simulink model "ClimaMgrForVentAndFlrDistbn"  (R2022b)
settings: Solver=FixedStepDiscrete  StartTime=0.0  StopTime=10.0  FixedStep=1  RelTol=1e-3
(part 1 of 2)
settings Model: Creator=blue.ge  LastModifiedBy=blue.ge
settings DataIOCC: SaveFormat=StructureWithTime
settings OptimizationCC: DefaultParameterBehavior=Inlined  EnhancedBackFolding=on  PassReuseOutputArgsAs=Individual arguments  ZeroExternalMemoryAtStartup=off  ZeroInternalMemoryAtStartup=off  OptimizeBlockOrder=speed  DifferentSizesBufferReuse=on  OptimizationCustomize=off  EfficientTunableParamExpr=on
settings DebuggingCC: ParameterTunabilityLossMsg=error
settings ModelReferenceCC: ModelReferenceNumInstancesAllowed=Single
settings SFSimCC: MATLABDynamicMemAlloc=off
settings RTWCC: Description=AUTOSAR  SystemTargetFile=autosar.tlc  GenCodeOnly=on  TemplateMakefile=ert_default_tmf
settings CodeAppCC: ForceParamTrailComments=on  IgnoreCustomStorageClasses=off  OperatorAnnotations=on  SimulinkDataObjDesc=on  SFDataObjDesc=on  InsertBlockDesc=on
settings STFCustomTargetCC: TargetLangStandard=C99 (ISO)  MultiwordLength=256  ParMdlRefBuildCompliant=on  CompOptLevelCompliant=on  IncludeMdlTerminateFcn=off  CombineOutputUpdateFcns=on  SuppressErrorStatus=on  ERTCustomFileBanners=on  SupportNonFinite=off  SupportComplex=off  SupportContinuousTime=off  SupportNonInlinedSFcns=off  RemoveResetFunc=on  MATLABClassNameForMDSCustomization=Simulink.SoftwareTarget.ERTCustomization  AutosarCompliant=on  UseToolchainInfoCompliant=on  LookupTableObjectStructAxisOrder=2,1,3,4,...  LUTObjectStructOrderEvenSpacing=Size,Table,Breakpoints  SystemTargetFile=autosar.tlc
settings ERTTargetCC: TargetLangStandard=C99 (ISO)  ConcurrentExecutionCompliant=off  IncludeMdlTerminateFcn=off  SuppressErrorStatus=on  SupportNonFinite=off  SupportComplex=off  ModelStepFunctionPrototypeControlCompliant=off  CPPClassGenCompliant=off  AutosarCompliant=on  LookupTableObjectStructAxisOrder=2,1,3,4,...  LUTObjectStructOrderEvenSpacing=Size,Table,Breakpoints  InlinedParameterPlacement=NonHierarchical  ERTCustomFileTemplate=example_file_process.tlc

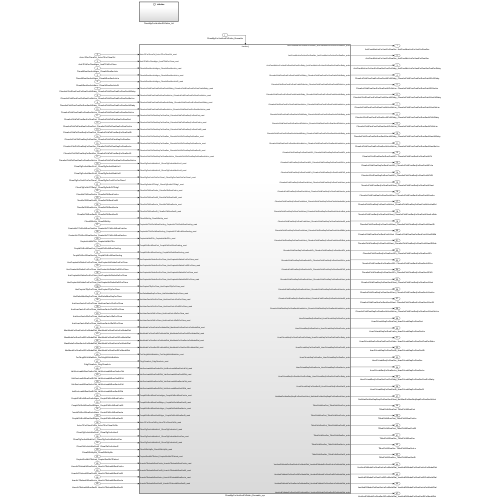
[thumbnail: Simulink's stored preview of the top-level diagram]
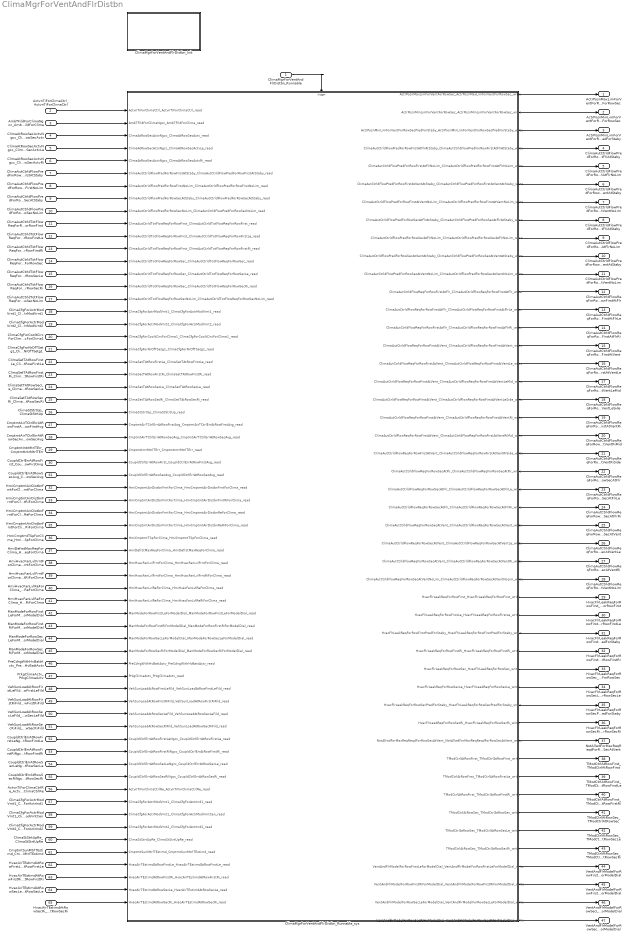
== system ClimaMgrForVentAndFlrDistbn ==
  ClimaMgrForVentAndFlrDistbn_Runnable : Inport  SampleTime=1  OutputFunctionCall=on
  ActvnTiForClimaCtrl_ActvnTiForClimaCtrl : Inport  Port=2  OutDataTypeStr=Ti33  PortDimensions=1
  AmbTFildForClimaNgcc_AmbTFildForClima : Inport  Port=3  OutDataTypeStr=T30  PortDimensions=1
  ClimaAtRowSecActvNgcc_ClimaAtRowSecActv : Inport  Port=4  OutDataTypeStr=Boolean  PortDimensions=1
  ClimaAtRowSecActvNgcc_ClimaAtRowSecActvLe : Inport  Port=5  OutDataTypeStr=Boolean  PortDimensions=1
  ClimaAtRowSecActvNgcc_ClimaAtRowSecActvRi : Inport  Port=6  OutDataTypeStr=Boolean  PortDimensions=1
  ClimaAutCtrldFlowPredForRowFirstAtStaby_ClimaAutCtrldFlowPredForRowFirstAtStaby : Inport  Port=7  OutDataTypeStr=AirFlow30  PortDimensions=1
  ClimaAutCtrldFlowPredForRowFirstNoLim_ClimaAutCtrldFlowPredForRowFirstNoLim : Inport  Port=8  OutDataTypeStr=AirFlow30  PortDimensions=1
  ClimaAutCtrldFlowPredForRowSecAtStaby_ClimaAutCtrldFlowPredForRowSecAtStaby : Inport  Port=9  OutDataTypeStr=AirFlow30  PortDimensions=1
  ClimaAutCtrldFlowPredForRowSecNoLim_ClimaAutCtrldFlowPredForRowSecNoLim : Inport  Port=10  OutDataTypeStr=AirFlow30  PortDimensions=1
  ClimaAutCtrldTotFlowReqForRowFirst_ClimaAutCtrldTotFlowReqForRowFirst : Inport  Port=11  OutDataTypeStr=AirFlow30  PortDimensions=1
  ClimaAutCtrldTotFlowReqForRowFirst_ClimaAutCtrldTotFlowReqForRowFirstLe : Inport  Port=12  OutDataTypeStr=AirFlow30  PortDimensions=1
  ClimaAutCtrldTotFlowReqForRowFirst_ClimaAutCtrldTotFlowReqForRowFirstRi : Inport  Port=13  OutDataTypeStr=AirFlow30  PortDimensions=1
  ClimaAutCtrldTotFlowReqForRowSec_ClimaAutCtrldTotFlowReqForRowSec : Inport  Port=14  OutDataTypeStr=AirFlow30  PortDimensions=1
  ClimaAutCtrldTotFlowReqForRowSec_ClimaAutCtrldTotFlowReqForRowSecLe : Inport  Port=15  OutDataTypeStr=AirFlow30  PortDimensions=1
  ClimaAutCtrldTotFlowReqForRowSec_ClimaAutCtrldTotFlowReqForRowSecRi : Inport  Port=16  OutDataTypeStr=AirFlow30  PortDimensions=1
  ClimaAutCtrldTotFlowReqForRowSecNoLim_ClimaAutCtrldTotFlowReqForRowSecNoLim : Inport  Port=17  OutDataTypeStr=AirFlow30  PortDimensions=1
  ClimaCfgForActrModVrnt1_ClimaCfgForActrModVrnt1 : Inport  Port=18  OutDataTypeStr=Enum: ClimaCfgForActrModVrnt1  PortDimensions=1
  ClimaCfgForActrModVrnt2_ClimaCfgForActrModVrnt2 : Inport  Port=19  OutDataTypeStr=Enum: ClimaCfgForActrModVrnt2  PortDimensions=1
  ClimaCfgForCooltCircForClima1_ClimaCfgForCooltCircForClima1 : Inport  Port=20  OutDataTypeStr=Boolean  PortDimensions=1
  ClimaCfgForNrOfTSetg1_ClimaCfgForNrOfTSetg1 : Inport  Port=21  OutDataTypeStr=Enum: ClimaCfgForNrOfTSetg1  PortDimensions=1
  ClimaSetTAtRowFirstLe_ClimaSetTAtRowFirstLe : Inport  Port=22  OutDataTypeStr=T30  PortDimensions=1
  ClimaSetTAtRowFirstRi_ClimaSetTAtRowFirstRi : Inport  Port=23  OutDataTypeStr=T30  PortDimensions=1
  ClimaSetTAtRowSecLe_ClimaSetTAtRowSecLe : Inport  Port=24  OutDataTypeStr=T30  PortDimensions=1
  ClimaSetTAtRowSecRi_ClimaSetTAtRowSecRi : Inport  Port=25  OutDataTypeStr=T30  PortDimensions=1
  ClimaStStrtUp_ClimaStStrtUp : Inport  Port=26  OutDataTypeStr=Enum: ClimaStStrtUp  PortDimensions=1
  CmptmtAirTCtrlErrAtRowFirstAvg_CmptmtAirTCtrlErrAtRowFirstAvg : Inport  Port=27  OutDataTypeStr=T30  PortDimensions=1
  CmptmtAirTCtrlErrAtRowSecAvg_CmptmtAirTCtrlErrAtRowSecAvg : Inport  Port=28  OutDataTypeStr=T30  PortDimensions=1
  CmptmtIntrMtrlTErr_CmptmtIntrMtrlTErr : Inport  Port=29  OutDataTypeStr=T30  PortDimensions=1
  CoupldCtrlErrAtRowFirst_CoupldCtrlErrAtRowFirstAvg : Inport  Port=30  OutDataTypeStr=T30  PortDimensions=1
  CoupldCtrlErrAtRowSecAvg_CoupldCtrlErrAtRowSecAvg : Inport  Port=31  OutDataTypeStr=T30  PortDimensions=1
  HmiCmptmtAirDistbnFrntForClima_HmiCmptmtAirDistbnFrntForClima : Inport  Port=32  OutDataTypeStr=Enum: HmiCmptmtAirDistbnFrnt  PortDimensions=1
  HmiCmptmtAirDistbnFrntForClima_HmiCmptmtAirDistbnFrntRiForClima : Inport  Port=33  OutDataTypeStr=Enum: HmiCmptmtAirDistbnFrnt  PortDimensions=1
  HmiCmptmtAirDistbnFrntForClima_HmiCmptmtAirDistbnReForClima : Inport  Port=34  OutDataTypeStr=Enum: HmiCmptmtAirDistbnFrnt  PortDimensions=1
  HmiCmptmtAirDistbnFrntForClima_HmiCmptmtAirDistbnReRiForClima : Inport  Port=35  OutDataTypeStr=Enum: HmiCmptmtAirDistbnFrnt  PortDimensions=1
  HmiCmptmtTSpForClima_HmiCmptmtTSpForClima : Inport  Port=36  OutDataTypeStr=Bus: HmiCmptmtTSp  PortDimensions=1  BusOutputAsStruct=on
  HmiDefrstMaxReqForClima_HmiDefrstMaxReqForClima : Inport  Port=37  OutDataTypeStr=Enum: ActrReq  PortDimensions=1
  HmiHvacFanLvlFrntForClima_HmiHvacFanLvlFrntForClima : Inport  Port=38  OutDataTypeStr=Enum: HmiHvacFanLvl  PortDimensions=1
  HmiHvacFanLvlFrntForClima_HmiHvacFanLvlFrntRiForClima : Inport  Port=39  OutDataTypeStr=Enum: HmiHvacFanLvl  PortDimensions=1
  HmiHvacFanLvlReForClima_HmiHvacFanLvlReForClima : Inport  Port=40  OutDataTypeStr=Enum: HmiHvacFanLvl  PortDimensions=1
  HmiHvacFanLvlReForClima_HmiHvacFanLvlReRiForClima : Inport  Port=41  OutDataTypeStr=Enum: HmiHvacFanLvl  PortDimensions=1
  ManModeForRowFirstLeForModelDial_ManModeForRowFirstLeForModelDial : Inport  Port=42  OutDataTypeStr=Enum: HmiCmptmtAirDistbnFrnt  PortDimensions=1
  ManModeForRowFirstRiForModelDial_ManModeForRowFirstRiForModelDial : Inport  Port=43  OutDataTypeStr=Enum: HmiCmptmtAirDistbnFrnt  PortDimensions=1
  ManModeForRowSecLeForModelDial_ManModeForRowSecLeForModelDial : Inport  Port=44  OutDataTypeStr=Enum: HmiCmptmtAirDistbnFrnt  PortDimensions=1
  ManModeForRowSecRiForModelDial_ManModeForRowSecRiForModelDial : Inport  Port=45  OutDataTypeStr=Enum: HmiCmptmtAirDistbnFrnt  PortDimensions=1
  PreCdngWithHvBattActv_PreCdngWithHvBattActv : Inport  Port=46  OutDataTypeStr=Boolean  PortDimensions=1
  PrkgClimaActv_PrkgClimaActv : Inport  Port=47  OutDataTypeStr=Enum: PrkgClimaActv  PortDimensions=1
  VehSunLoadAtRowFirstLeFild_VehSunLoadAtRowFirstLeFild : Inport  Port=48  OutDataTypeStr=SunLoad30  PortDimensions=1
  VehSunLoadAtRowFirstRiFild_VehSunLoadAtRowFirstRiFild : Inport  Port=49  OutDataTypeStr=SunLoad30  PortDimensions=1
  VehSunLoadAtRowSecLeFild_VehSunLoadAtRowSecLeFild : Inport  Port=50  OutDataTypeStr=SunLoad30  PortDimensions=1
  VehSunLoadAtRowSecRiFild_VehSunLoadAtRowSecRiFild : Inport  Port=51  OutDataTypeStr=SunLoad30  PortDimensions=1
  CoupldCtrlErrAtRowFirstLeNgcc_CoupldCtrlErrAtRowFirstLe : Inport  Port=52  OutDataTypeStr=T30  PortDimensions=1
  CoupldCtrlErrAtRowFirstRiNgcc_CoupldCtrlErrAtRowFirstRi : Inport  Port=53  OutDataTypeStr=T30  PortDimensions=1
  CoupldCtrlErrAtRowSecLeNgcc_CoupldCtrlErrAtRowSecLe : Inport  Port=54  OutDataTypeStr=T30  PortDimensions=1
  CoupldCtrlErrAtRowSecRiNgcc_CoupldCtrlErrAtRowSecRi : Inport  Port=55  OutDataTypeStr=T30  PortDimensions=1
  ActvnTiForClimaCtrlRe_ActvnTiForClimaCtrlRe : Inport  Port=56  OutDataTypeStr=Ti33  PortDimensions=1
  ClimaCfgForActrModVrnt1_ClimaCfgForActrInd1 : Inport  Port=57  OutDataTypeStr=Enum: ClimaCfgForActrInd1  PortDimensions=1
  ClimaCfgForActrModVrnt1_ClimaCfgForActrModVrntCen : Inport  Port=58  OutDataTypeStr=Enum: ClimaCfgForActrModVrntCen  PortDimensions=1
  ClimaCfgForActrModVrnt2_ClimaCfgForActrInd2 : Inport  Port=59  OutDataTypeStr=Enum: ClimaCfgForActrInd2  PortDimensions=1
  ClimaStStrtUpRe_ClimaStStrtUpRe : Inport  Port=60  OutDataTypeStr=Enum: ClimaStStrtUp  PortDimensions=1
  CmptmtSunMtrlTEstimd_CmptmtSunMtrlTEstimd : Inport  Port=61  OutDataTypeStr=T30  PortDimensions=1
  HvacAirTEstimdAtRowFirstLe_HvacAirTEstimdAtRowFirstLe : Inport  Port=62  OutDataTypeStr=T30  PortDimensions=1
  HvacAirTEstimdAtRowFirstRi_HvacAirTEstimdAtRowFirstRi : Inport  Port=63  OutDataTypeStr=T30  PortDimensions=1
  HvacAirTEstimdAtRowSecLe_HvacAirTEstimdAtRowSecLe : Inport  Port=64  OutDataTypeStr=T30  PortDimensions=1
  HvacAirTEstimdAtRowSecRi_HvacAirTEstimdAtRowSecRi : Inport  Port=65  OutDataTypeStr=T30  PortDimensions=1
  ClimaMgrForVentAndFlrDistbn_Init : Subsystem
  ClimaMgrForVentAndFlrDistbn_Runnable_sys : Subsystem
  ActrPosnMaxLimForVentForRowSec_ActrPosnMaxLimForVentForRowSec : Outport  OutDataTypeStr=Perc30  PortDimensions=1
  ActrPosnMinLimForVentForRowSec_ActrPosnMinLimForVentForRowSec : Outport  Port=2  OutDataTypeStr=Perc30  PortDimensions=1
  ActrPosnMinLimForVentForRowSecPredForStaby_ActrPosnMinLimForVentForRowSecPredForStaby : Outport  Port=3  OutDataTypeStr=Perc30  PortDimensions=1
  ClimaAutCtrldFlowPredForRowFirstAtFlrAtStaby_ClimaAutCtrldFlowPredForRowFirstAtFlrAtStaby : Outport  Port=4  OutDataTypeStr=AirFlow30  PortDimensions=1
  ClimaAutCtrldFlowPredForRowFirstAtFlrNoLim_ClimaAutCtrldFlowPredForRowFirstAtFlrNoLim : Outport  Port=5  OutDataTypeStr=AirFlow30  PortDimensions=1
  ClimaAutCtrldFlowPredForRowFirstAtVentAtStaby_ClimaAutCtrldFlowPredForRowFirstAtVentAtStaby : Outport  Port=6  OutDataTypeStr=AirFlow30  PortDimensions=1
  ClimaAutCtrldFlowPredForRowFirstAtVentNoLim_ClimaAutCtrldFlowPredForRowFirstAtVentNoLim : Outport  Port=7  OutDataTypeStr=AirFlow30  PortDimensions=1
  ClimaAutCtrldFlowPredForRowSecAtFlrAtStaby_ClimaAutCtrldFlowPredForRowSecAtFlrAtStaby : Outport  Port=8  OutDataTypeStr=AirFlow30  PortDimensions=1
  ClimaAutCtrldFlowPredForRowSecAtFlrNoLim_ClimaAutCtrldFlowPredForRowSecAtFlrNoLim : Outport  Port=9  OutDataTypeStr=AirFlow30  PortDimensions=1
  ClimaAutCtrldFlowPredForRowSecAtVentAtStaby_ClimaAutCtrldFlowPredForRowSecAtVentAtStaby : Outport  Port=10  OutDataTypeStr=AirFlow30  PortDimensions=1
  ClimaAutCtrldFlowPredForRowSecAtVentNoLim_ClimaAutCtrldFlowPredForRowSecAtVentNoLim : Outport  Port=11  OutDataTypeStr=AirFlow30  PortDimensions=1
  ClimaAutCtrldFlowReqForRowFirstAtFlr_ClimaAutCtrldFlowReqForRowFirstAtFlr : Outport  Port=12  OutDataTypeStr=AirFlow30  PortDimensions=1
  ClimaAutCtrldFlowReqForRowFirstAtFlr_ClimaAutCtrldFlowReqForRowFirstAtFlrLe : Outport  Port=13  OutDataTypeStr=AirFlow30  PortDimensions=1
  ClimaAutCtrldFlowReqForRowFirstAtFlr_ClimaAutCtrldFlowReqForRowFirstAtFlrRi : Outport  Port=14  OutDataTypeStr=AirFlow30  PortDimensions=1
  ClimaAutCtrldFlowReqForRowFirstAtVent_ClimaAutCtrldFlowReqForRowFirstAtVent : Outport  Port=15  OutDataTypeStr=AirFlow30  PortDimensions=1
  ClimaAutCtrldFlowReqForRowFirstAtVent_ClimaAutCtrldFlowReqForRowFirstAtVentLe : Outport  Port=16  OutDataTypeStr=AirFlow30  PortDimensions=1
  ClimaAutCtrldFlowReqForRowFirstAtVent_ClimaAutCtrldFlowReqForRowFirstAtVentLeMid : Outport  Port=17  OutDataTypeStr=AirFlow30  PortDimensions=1
  ClimaAutCtrldFlowReqForRowFirstAtVent_ClimaAutCtrldFlowReqForRowFirstAtVentLeSide : Outport  Port=18  OutDataTypeStr=AirFlow30  PortDimensions=1
  ClimaAutCtrldFlowReqForRowFirstAtVent_ClimaAutCtrldFlowReqForRowFirstAtVentRi : Outport  Port=19  OutDataTypeStr=AirFlow30  PortDimensions=1
  ClimaAutCtrldFlowReqForRowFirstAtVent_ClimaAutCtrldFlowReqForRowFirstAtVentRiMid : Outport  Port=20  OutDataTypeStr=AirFlow30  PortDimensions=1
  ClimaAutCtrldFlowReqForRowFirstAtVent_ClimaAutCtrldFlowReqForRowFirstAtVentRiSide : Outport  Port=21  OutDataTypeStr=AirFlow30  PortDimensions=1
  ClimaAutCtrldFlowReqForRowSecAtFlr_ClimaAutCtrldFlowReqForRowSecAtFlr : Outport  Port=22  OutDataTypeStr=AirFlow30  PortDimensions=1
  ClimaAutCtrldFlowReqForRowSecAtFlr_ClimaAutCtrldFlowReqForRowSecAtFlrLe : Outport  Port=23  OutDataTypeStr=AirFlow30  PortDimensions=1
  ClimaAutCtrldFlowReqForRowSecAtFlr_ClimaAutCtrldFlowReqForRowSecAtFlrRi : Outport  Port=24  OutDataTypeStr=AirFlow30  PortDimensions=1
  ClimaAutCtrldFlowReqForRowSecAtVent_ClimaAutCtrldFlowReqForRowSecAtVent : Outport  Port=25  OutDataTypeStr=AirFlow30  PortDimensions=1
  ClimaAutCtrldFlowReqForRowSecAtVent_ClimaAutCtrldFlowReqForRowSecAtVentLe : Outport  Port=26  OutDataTypeStr=AirFlow30  PortDimensions=1
  ClimaAutCtrldFlowReqForRowSecAtVent_ClimaAutCtrldFlowReqForRowSecAtVentRi : Outport  Port=27  OutDataTypeStr=AirFlow30  PortDimensions=1
  ClimaAutCtrldFlowReqForRowSecAtVentNoLim_ClimaAutCtrldFlowReqForRowSecAtVentNoLim : Outport  Port=28  OutDataTypeStr=AirFlow30  PortDimensions=1
  HvacFlrLeakReqForRowFirst_HvacFlrLeakReqForRowFirst : Outport  Port=29  OutDataTypeStr=Boolean  PortDimensions=1
  HvacFlrLeakReqForRowFirstLe_HvacFlrLeakReqForRowFirstLe : Outport  Port=30  OutDataTypeStr=Boolean  PortDimensions=1
  HvacFlrLeakReqForRowFirstPredForStaby_HvacFlrLeakReqForRowFirstPredForStaby : Outport  Port=31  OutDataTypeStr=Boolean  PortDimensions=1
  HvacFlrLeakReqForRowFirstRi_HvacFlrLeakReqForRowFirstRi : Outport  Port=32  OutDataTypeStr=Boolean  PortDimensions=1
  HvacFlrLeakReqForRowSec_HvacFlrLeakReqForRowSec : Outport  Port=33  OutDataTypeStr=Boolean  PortDimensions=1
  HvacFlrLeakReqForRowSecLe_HvacFlrLeakReqForRowSecLe : Outport  Port=34  OutDataTypeStr=Boolean  PortDimensions=1
  HvacFlrLeakReqForRowSecPredForStaby_HvacFlrLeakReqForRowSecPredForStaby : Outport  Port=35  OutDataTypeStr=Boolean  PortDimensions=1
  HvacFlrLeakReqForRowSecRi_HvacFlrLeakReqForRowSecRi : Outport  Port=36  OutDataTypeStr=Boolean  PortDimensions=1
  NotAllwdForMaxReqReqdForRowSecAtVent_NotAllwdForMaxReqReqdForRowSecAtVent : Outport  Port=37  OutDataTypeStr=Boolean  PortDimensions=1
  TModCtrlAtRowFirst_TModCtrlAtRowFirst : Outport  Port=38  OutDataTypeStr=T31  PortDimensions=1
  TModCtrlAtRowFirst_TModCtrlAtRowFirstLe : Outport  Port=39  OutDataTypeStr=T31  PortDimensions=1
  TModCtrlAtRowFirst_TModCtrlAtRowFirstRi : Outport  Port=40  OutDataTypeStr=T31  PortDimensions=1
  TModCtrlAtRowSec_TModCtrlAtRowSec : Outport  Port=41  OutDataTypeStr=T31  PortDimensions=1
  TModCtrlAtRowSec_TModCtrlAtRowSecLe : Outport  Port=42  OutDataTypeStr=T31  PortDimensions=1
  TModCtrlAtRowSec_TModCtrlAtRowSecRi : Outport  Port=43  OutDataTypeStr=T31  PortDimensions=1
  VentAndFlrModelForRowFirstLeForModelDial_VentAndFlrModelForRowFirstLeForModelDial : Outport  Port=44  OutDataTypeStr=Enum: HmiCmptmtAirDistbnFrnt  PortDimensions=1
  VentAndFlrModelForRowFirstRiForModelDial_VentAndFlrModelForRowFirstRiForModelDial : Outport  Port=45  OutDataTypeStr=Enum: HmiCmptmtAirDistbnFrnt  PortDimensions=1
  VentAndFlrModelForRowSecLeForModelDial_VentAndFlrModelForRowSecLeForModelDial : Outport  Port=46  OutDataTypeStr=Enum: HmiCmptmtAirDistbnFrnt  PortDimensions=1
  VentAndFlrModelForRowSecRiForModelDial_VentAndFlrModelForRowSecRiForModelDial : Outport  Port=47  OutDataTypeStr=Enum: HmiCmptmtAirDistbnFrnt  PortDimensions=1
  wires:
  ActvnTiForClimaCtrl_ActvnTiForClimaCtrl/1 -> ClimaMgrForVentAndFlrDistbn_Runnable_sys/1(ActvnTiForClimaCtrl_ActvnTiForClimaCtrl_read)
  AmbTFildForClimaNgcc_AmbTFildForClima/1 -> ClimaMgrForVentAndFlrDistbn_Runnable_sys/2(AmbTFildForClimaNgcc_AmbTFildForClima_read)
  ClimaAtRowSecActvNgcc_ClimaAtRowSecActv/1 -> ClimaMgrForVentAndFlrDistbn_Runnable_sys/3(ClimaAtRowSecActvNgcc_ClimaAtRowSecActv_read)
  ClimaAtRowSecActvNgcc_ClimaAtRowSecActvLe/1 -> ClimaMgrForVentAndFlrDistbn_Runnable_sys/4(ClimaAtRowSecActvNgcc_ClimaAtRowSecActvLe_read)
  ClimaAtRowSecActvNgcc_ClimaAtRowSecActvRi/1 -> ClimaMgrForVentAndFlrDistbn_Runnable_sys/5(ClimaAtRowSecActvNgcc_ClimaAtRowSecActvRi_read)
  ClimaAutCtrldFlowPredForRowFirstAtStaby_ClimaAutCtrldFlowPredForRowFirstAtStaby/1 -> ClimaMgrForVentAndFlrDistbn_Runnable_sys/6(ClimaAutCtrldFlowPredForRowFirstAtStaby_ClimaAutCtrldFlowPredForRowFirstAtStaby_read)
  ClimaAutCtrldFlowPredForRowFirstNoLim_ClimaAutCtrldFlowPredForRowFirstNoLim/1 -> ClimaMgrForVentAndFlrDistbn_Runnable_sys/7(ClimaAutCtrldFlowPredForRowFirstNoLim_ClimaAutCtrldFlowPredForRowFirstNoLim_read)
  ClimaAutCtrldFlowPredForRowSecAtStaby_ClimaAutCtrldFlowPredForRowSecAtStaby/1 -> ClimaMgrForVentAndFlrDistbn_Runnable_sys/8(ClimaAutCtrldFlowPredForRowSecAtStaby_ClimaAutCtrldFlowPredForRowSecAtStaby_read)
  ClimaAutCtrldFlowPredForRowSecNoLim_ClimaAutCtrldFlowPredForRowSecNoLim/1 -> ClimaMgrForVentAndFlrDistbn_Runnable_sys/9(ClimaAutCtrldFlowPredForRowSecNoLim_ClimaAutCtrldFlowPredForRowSecNoLim_read)
  ClimaAutCtrldTotFlowReqForRowFirst_ClimaAutCtrldTotFlowReqForRowFirst/1 -> ClimaMgrForVentAndFlrDistbn_Runnable_sys/10(ClimaAutCtrldTotFlowReqForRowFirst_ClimaAutCtrldTotFlowReqForRowFirst_read)
  ClimaAutCtrldTotFlowReqForRowFirst_ClimaAutCtrldTotFlowReqForRowFirstLe/1 -> ClimaMgrForVentAndFlrDistbn_Runnable_sys/11(ClimaAutCtrldTotFlowReqForRowFirst_ClimaAutCtrldTotFlowReqForRowFirstLe_read)
  ClimaAutCtrldTotFlowReqForRowFirst_ClimaAutCtrldTotFlowReqForRowFirstRi/1 -> ClimaMgrForVentAndFlrDistbn_Runnable_sys/12(ClimaAutCtrldTotFlowReqForRowFirst_ClimaAutCtrldTotFlowReqForRowFirstRi_read)
  ClimaAutCtrldTotFlowReqForRowSec_ClimaAutCtrldTotFlowReqForRowSec/1 -> ClimaMgrForVentAndFlrDistbn_Runnable_sys/13(ClimaAutCtrldTotFlowReqForRowSec_ClimaAutCtrldTotFlowReqForRowSec_read)
  ClimaAutCtrldTotFlowReqForRowSec_ClimaAutCtrldTotFlowReqForRowSecLe/1 -> ClimaMgrForVentAndFlrDistbn_Runnable_sys/14(ClimaAutCtrldTotFlowReqForRowSec_ClimaAutCtrldTotFlowReqForRowSecLe_read)
  ClimaAutCtrldTotFlowReqForRowSec_ClimaAutCtrldTotFlowReqForRowSecRi/1 -> ClimaMgrForVentAndFlrDistbn_Runnable_sys/15(ClimaAutCtrldTotFlowReqForRowSec_ClimaAutCtrldTotFlowReqForRowSecRi_read)
  ClimaAutCtrldTotFlowReqForRowSecNoLim_ClimaAutCtrldTotFlowReqForRowSecNoLim/1 -> ClimaMgrForVentAndFlrDistbn_Runnable_sys/16(ClimaAutCtrldTotFlowReqForRowSecNoLim_ClimaAutCtrldTotFlowReqForRowSecNoLim_read)
  ClimaCfgForActrModVrnt1_ClimaCfgForActrModVrnt1/1 -> ClimaMgrForVentAndFlrDistbn_Runnable_sys/17(ClimaCfgForActrModVrnt1_ClimaCfgForActrModVrnt1_read)
  ClimaCfgForActrModVrnt2_ClimaCfgForActrModVrnt2/1 -> ClimaMgrForVentAndFlrDistbn_Runnable_sys/18(ClimaCfgForActrModVrnt2_ClimaCfgForActrModVrnt2_read)
  ClimaCfgForCooltCircForClima1_ClimaCfgForCooltCircForClima1/1 -> ClimaMgrForVentAndFlrDistbn_Runnable_sys/19(ClimaCfgForCooltCircForClima1_ClimaCfgForCooltCircForClima1_read)
  ClimaCfgForNrOfTSetg1_ClimaCfgForNrOfTSetg1/1 -> ClimaMgrForVentAndFlrDistbn_Runnable_sys/20(ClimaCfgForNrOfTSetg1_ClimaCfgForNrOfTSetg1_read)
  ClimaSetTAtRowFirstLe_ClimaSetTAtRowFirstLe/1 -> ClimaMgrForVentAndFlrDistbn_Runnable_sys/21(ClimaSetTAtRowFirstLe_ClimaSetTAtRowFirstLe_read)
  ClimaSetTAtRowFirstRi_ClimaSetTAtRowFirstRi/1 -> ClimaMgrForVentAndFlrDistbn_Runnable_sys/22(ClimaSetTAtRowFirstRi_ClimaSetTAtRowFirstRi_read)
  ClimaSetTAtRowSecLe_ClimaSetTAtRowSecLe/1 -> ClimaMgrForVentAndFlrDistbn_Runnable_sys/23(ClimaSetTAtRowSecLe_ClimaSetTAtRowSecLe_read)
  ClimaSetTAtRowSecRi_ClimaSetTAtRowSecRi/1 -> ClimaMgrForVentAndFlrDistbn_Runnable_sys/24(ClimaSetTAtRowSecRi_ClimaSetTAtRowSecRi_read)
  ClimaStStrtUp_ClimaStStrtUp/1 -> ClimaMgrForVentAndFlrDistbn_Runnable_sys/25(ClimaStStrtUp_ClimaStStrtUp_read)
  CmptmtAirTCtrlErrAtRowFirstAvg_CmptmtAirTCtrlErrAtRowFirstAvg/1 -> ClimaMgrForVentAndFlrDistbn_Runnable_sys/26(CmptmtAirTCtrlErrAtRowFirstAvg_CmptmtAirTCtrlErrAtRowFirstAvg_read)
  CmptmtAirTCtrlErrAtRowSecAvg_CmptmtAirTCtrlErrAtRowSecAvg/1 -> ClimaMgrForVentAndFlrDistbn_Runnable_sys/27(CmptmtAirTCtrlErrAtRowSecAvg_CmptmtAirTCtrlErrAtRowSecAvg_read)
  CmptmtIntrMtrlTErr_CmptmtIntrMtrlTErr/1 -> ClimaMgrForVentAndFlrDistbn_Runnable_sys/28(CmptmtIntrMtrlTErr_CmptmtIntrMtrlTErr_read)
  CoupldCtrlErrAtRowFirst_CoupldCtrlErrAtRowFirstAvg/1 -> ClimaMgrForVentAndFlrDistbn_Runnable_sys/29(CoupldCtrlErrAtRowFirst_CoupldCtrlErrAtRowFirstAvg_read)
  CoupldCtrlErrAtRowSecAvg_CoupldCtrlErrAtRowSecAvg/1 -> ClimaMgrForVentAndFlrDistbn_Runnable_sys/30(CoupldCtrlErrAtRowSecAvg_CoupldCtrlErrAtRowSecAvg_read)
  HmiCmptmtAirDistbnFrntForClima_HmiCmptmtAirDistbnFrntForClima/1 -> ClimaMgrForVentAndFlrDistbn_Runnable_sys/31(HmiCmptmtAirDistbnFrntForClima_HmiCmptmtAirDistbnFrntForClima_read)
  HmiCmptmtAirDistbnFrntForClima_HmiCmptmtAirDistbnFrntRiForClima/1 -> ClimaMgrForVentAndFlrDistbn_Runnable_sys/32(HmiCmptmtAirDistbnFrntForClima_HmiCmptmtAirDistbnFrntRiForClima_read)
  HmiCmptmtAirDistbnFrntForClima_HmiCmptmtAirDistbnReForClima/1 -> ClimaMgrForVentAndFlrDistbn_Runnable_sys/33(HmiCmptmtAirDistbnFrntForClima_HmiCmptmtAirDistbnReForClima_read)
  HmiCmptmtAirDistbnFrntForClima_HmiCmptmtAirDistbnReRiForClima/1 -> ClimaMgrForVentAndFlrDistbn_Runnable_sys/34(HmiCmptmtAirDistbnFrntForClima_HmiCmptmtAirDistbnReRiForClima_read)
  HmiCmptmtTSpForClima_HmiCmptmtTSpForClima/1 -> ClimaMgrForVentAndFlrDistbn_Runnable_sys/35(HmiCmptmtTSpForClima_HmiCmptmtTSpForClima_read)
  HmiDefrstMaxReqForClima_HmiDefrstMaxReqForClima/1 -> ClimaMgrForVentAndFlrDistbn_Runnable_sys/36(HmiDefrstMaxReqForClima_HmiDefrstMaxReqForClima_read)
  HmiHvacFanLvlFrntForClima_HmiHvacFanLvlFrntForClima/1 -> ClimaMgrForVentAndFlrDistbn_Runnable_sys/37(HmiHvacFanLvlFrntForClima_HmiHvacFanLvlFrntForClima_read)
  HmiHvacFanLvlFrntForClima_HmiHvacFanLvlFrntRiForClima/1 -> ClimaMgrForVentAndFlrDistbn_Runnable_sys/38(HmiHvacFanLvlFrntForClima_HmiHvacFanLvlFrntRiForClima_read)
  HmiHvacFanLvlReForClima_HmiHvacFanLvlReForClima/1 -> ClimaMgrForVentAndFlrDistbn_Runnable_sys/39(HmiHvacFanLvlReForClima_HmiHvacFanLvlReForClima_read)
  HmiHvacFanLvlReForClima_HmiHvacFanLvlReRiForClima/1 -> ClimaMgrForVentAndFlrDistbn_Runnable_sys/40(HmiHvacFanLvlReForClima_HmiHvacFanLvlReRiForClima_read)
  ManModeForRowFirstLeForModelDial_ManModeForRowFirstLeForModelDial/1 -> ClimaMgrForVentAndFlrDistbn_Runnable_sys/41(ManModeForRowFirstLeForModelDial_ManModeForRowFirstLeForModelDial_read)
  ManModeForRowFirstRiForModelDial_ManModeForRowFirstRiForModelDial/1 -> ClimaMgrForVentAndFlrDistbn_Runnable_sys/42(ManModeForRowFirstRiForModelDial_ManModeForRowFirstRiForModelDial_read)
  ManModeForRowSecLeForModelDial_ManModeForRowSecLeForModelDial/1 -> ClimaMgrForVentAndFlrDistbn_Runnable_sys/43(ManModeForRowSecLeForModelDial_ManModeForRowSecLeForModelDial_read)
  ManModeForRowSecRiForModelDial_ManModeForRowSecRiForModelDial/1 -> ClimaMgrForVentAndFlrDistbn_Runnable_sys/44(ManModeForRowSecRiForModelDial_ManModeForRowSecRiForModelDial_read)
  PreCdngWithHvBattActv_PreCdngWithHvBattActv/1 -> ClimaMgrForVentAndFlrDistbn_Runnable_sys/45(PreCdngWithHvBattActv_PreCdngWithHvBattActv_read)
  PrkgClimaActv_PrkgClimaActv/1 -> ClimaMgrForVentAndFlrDistbn_Runnable_sys/46(PrkgClimaActv_PrkgClimaActv_read)
  VehSunLoadAtRowFirstLeFild_VehSunLoadAtRowFirstLeFild/1 -> ClimaMgrForVentAndFlrDistbn_Runnable_sys/47(VehSunLoadAtRowFirstLeFild_VehSunLoadAtRowFirstLeFild_read)
  VehSunLoadAtRowFirstRiFild_VehSunLoadAtRowFirstRiFild/1 -> ClimaMgrForVentAndFlrDistbn_Runnable_sys/48(VehSunLoadAtRowFirstRiFild_VehSunLoadAtRowFirstRiFild_read)
  VehSunLoadAtRowSecLeFild_VehSunLoadAtRowSecLeFild/1 -> ClimaMgrForVentAndFlrDistbn_Runnable_sys/49(VehSunLoadAtRowSecLeFild_VehSunLoadAtRowSecLeFild_read)
  VehSunLoadAtRowSecRiFild_VehSunLoadAtRowSecRiFild/1 -> ClimaMgrForVentAndFlrDistbn_Runnable_sys/50(VehSunLoadAtRowSecRiFild_VehSunLoadAtRowSecRiFild_read)
  CoupldCtrlErrAtRowFirstLeNgcc_CoupldCtrlErrAtRowFirstLe/1 -> ClimaMgrForVentAndFlrDistbn_Runnable_sys/51(CoupldCtrlErrAtRowFirstLeNgcc_CoupldCtrlErrAtRowFirstLe_read)
  CoupldCtrlErrAtRowFirstRiNgcc_CoupldCtrlErrAtRowFirstRi/1 -> ClimaMgrForVentAndFlrDistbn_Runnable_sys/52(CoupldCtrlErrAtRowFirstRiNgcc_CoupldCtrlErrAtRowFirstRi_read)
  CoupldCtrlErrAtRowSecLeNgcc_CoupldCtrlErrAtRowSecLe/1 -> ClimaMgrForVentAndFlrDistbn_Runnable_sys/53(CoupldCtrlErrAtRowSecLeNgcc_CoupldCtrlErrAtRowSecLe_read)
  CoupldCtrlErrAtRowSecRiNgcc_CoupldCtrlErrAtRowSecRi/1 -> ClimaMgrForVentAndFlrDistbn_Runnable_sys/54(CoupldCtrlErrAtRowSecRiNgcc_CoupldCtrlErrAtRowSecRi_read)
  ActvnTiForClimaCtrlRe_ActvnTiForClimaCtrlRe/1 -> ClimaMgrForVentAndFlrDistbn_Runnable_sys/55(ActvnTiForClimaCtrlRe_ActvnTiForClimaCtrlRe_read)
  ClimaCfgForActrModVrnt1_ClimaCfgForActrInd1/1 -> ClimaMgrForVentAndFlrDistbn_Runnable_sys/56(ClimaCfgForActrModVrnt1_ClimaCfgForActrInd1_read)
  ClimaCfgForActrModVrnt1_ClimaCfgForActrModVrntCen/1 -> ClimaMgrForVentAndFlrDistbn_Runnable_sys/57(ClimaCfgForActrModVrnt1_ClimaCfgForActrModVrntCen_read)
  ClimaCfgForActrModVrnt2_ClimaCfgForActrInd2/1 -> ClimaMgrForVentAndFlrDistbn_Runnable_sys/58(ClimaCfgForActrModVrnt2_ClimaCfgForActrInd2_read)
  ClimaStStrtUpRe_ClimaStStrtUpRe/1 -> ClimaMgrForVentAndFlrDistbn_Runnable_sys/59(ClimaStStrtUpRe_ClimaStStrtUpRe_read)
  CmptmtSunMtrlTEstimd_CmptmtSunMtrlTEstimd/1 -> ClimaMgrForVentAndFlrDistbn_Runnable_sys/60(CmptmtSunMtrlTEstimd_CmptmtSunMtrlTEstimd_read)
  HvacAirTEstimdAtRowFirstLe_HvacAirTEstimdAtRowFirstLe/1 -> ClimaMgrForVentAndFlrDistbn_Runnable_sys/61(HvacAirTEstimdAtRowFirstLe_HvacAirTEstimdAtRowFirstLe_read)
  HvacAirTEstimdAtRowFirstRi_HvacAirTEstimdAtRowFirstRi/1 -> ClimaMgrForVentAndFlrDistbn_Runnable_sys/62(HvacAirTEstimdAtRowFirstRi_HvacAirTEstimdAtRowFirstRi_read)
  HvacAirTEstimdAtRowSecLe_HvacAirTEstimdAtRowSecLe/1 -> ClimaMgrForVentAndFlrDistbn_Runnable_sys/63(HvacAirTEstimdAtRowSecLe_HvacAirTEstimdAtRowSecLe_read)
  HvacAirTEstimdAtRowSecRi_HvacAirTEstimdAtRowSecRi/1 -> ClimaMgrForVentAndFlrDistbn_Runnable_sys/64(HvacAirTEstimdAtRowSecRi_HvacAirTEstimdAtRowSecRi_read)
  ClimaMgrForVentAndFlrDistbn_Runnable_sys/1(ActrPosnMaxLimForVentForRowSec_ActrPosnMaxLimForVentForRowSec_write) -> ActrPosnMaxLimForVentForRowSec_ActrPosnMaxLimForVentForRowSec/1
  ClimaMgrForVentAndFlrDistbn_Runnable_sys/2(ActrPosnMinLimForVentForRowSec_ActrPosnMinLimForVentForRowSec_write) -> ActrPosnMinLimForVentForRowSec_ActrPosnMinLimForVentForRowSec/1
  ClimaMgrForVentAndFlrDistbn_Runnable_sys/3(ActrPosnMinLimForVentForRowSecPredForStaby_ActrPosnMinLimForVentForRowSecPredForStaby_write) -> ActrPosnMinLimForVentForRowSecPredForStaby_ActrPosnMinLimForVentForRowSecPredForStaby/1
  ClimaMgrForVentAndFlrDistbn_Runnable_sys/4(ClimaAutCtrldFlowPredForRowFirstAtFlrAtStaby_ClimaAutCtrldFlowPredForRowFirstAtFlrAtStaby_write) -> ClimaAutCtrldFlowPredForRowFirstAtFlrAtStaby_ClimaAutCtrldFlowPredForRowFirstAtFlrAtStaby/1
  ClimaMgrForVentAndFlrDistbn_Runnable_sys/5(ClimaAutCtrldFlowPredForRowFirstAtFlrNoLim_ClimaAutCtrldFlowPredForRowFirstAtFlrNoLim_write) -> ClimaAutCtrldFlowPredForRowFirstAtFlrNoLim_ClimaAutCtrldFlowPredForRowFirstAtFlrNoLim/1
  ClimaMgrForVentAndFlrDistbn_Runnable_sys/6(ClimaAutCtrldFlowPredForRowFirstAtVentAtStaby_ClimaAutCtrldFlowPredForRowFirstAtVentAtStaby_write) -> ClimaAutCtrldFlowPredForRowFirstAtVentAtStaby_ClimaAutCtrldFlowPredForRowFirstAtVentAtStaby/1
  ClimaMgrForVentAndFlrDistbn_Runnable_sys/7(ClimaAutCtrldFlowPredForRowFirstAtVentNoLim_ClimaAutCtrldFlowPredForRowFirstAtVentNoLim_write) -> ClimaAutCtrldFlowPredForRowFirstAtVentNoLim_ClimaAutCtrldFlowPredForRowFirstAtVentNoLim/1
  ClimaMgrForVentAndFlrDistbn_Runnable_sys/8(ClimaAutCtrldFlowPredForRowSecAtFlrAtStaby_ClimaAutCtrldFlowPredForRowSecAtFlrAtStaby_write) -> ClimaAutCtrldFlowPredForRowSecAtFlrAtStaby_ClimaAutCtrldFlowPredForRowSecAtFlrAtStaby/1
  ClimaMgrForVentAndFlrDistbn_Runnable_sys/9(ClimaAutCtrldFlowPredForRowSecAtFlrNoLim_ClimaAutCtrldFlowPredForRowSecAtFlrNoLim_write) -> ClimaAutCtrldFlowPredForRowSecAtFlrNoLim_ClimaAutCtrldFlowPredForRowSecAtFlrNoLim/1
  ClimaMgrForVentAndFlrDistbn_Runnable_sys/10(ClimaAutCtrldFlowPredForRowSecAtVentAtStaby_ClimaAutCtrldFlowPredForRowSecAtVentAtStaby_write) -> ClimaAutCtrldFlowPredForRowSecAtVentAtStaby_ClimaAutCtrldFlowPredForRowSecAtVentAtStaby/1
  ClimaMgrForVentAndFlrDistbn_Runnable_sys/11(ClimaAutCtrldFlowPredForRowSecAtVentNoLim_ClimaAutCtrldFlowPredForRowSecAtVentNoLim_write) -> ClimaAutCtrldFlowPredForRowSecAtVentNoLim_ClimaAutCtrldFlowPredForRowSecAtVentNoLim/1
  ClimaMgrForVentAndFlrDistbn_Runnable_sys/12(ClimaAutCtrldFlowReqForRowFirstAtFlr_ClimaAutCtrldFlowReqForRowFirstAtFlr_write) -> ClimaAutCtrldFlowReqForRowFirstAtFlr_ClimaAutCtrldFlowReqForRowFirstAtFlr/1
  ClimaMgrForVentAndFlrDistbn_Runnable_sys/13(ClimaAutCtrldFlowReqForRowFirstAtFlr_ClimaAutCtrldFlowReqForRowFirstAtFlrLe_write) -> ClimaAutCtrldFlowReqForRowFirstAtFlr_ClimaAutCtrldFlowReqForRowFirstAtFlrLe/1
  ClimaMgrForVentAndFlrDistbn_Runnable_sys/14(ClimaAutCtrldFlowReqForRowFirstAtFlr_ClimaAutCtrldFlowReqForRowFirstAtFlrRi_write) -> ClimaAutCtrldFlowReqForRowFirstAtFlr_ClimaAutCtrldFlowReqForRowFirstAtFlrRi/1
  ClimaMgrForVentAndFlrDistbn_Runnable_sys/15(ClimaAutCtrldFlowReqForRowFirstAtVent_ClimaAutCtrldFlowReqForRowFirstAtVent_write) -> ClimaAutCtrldFlowReqForRowFirstAtVent_ClimaAutCtrldFlowReqForRowFirstAtVent/1
  ClimaMgrForVentAndFlrDistbn_Runnable_sys/16(ClimaAutCtrldFlowReqForRowFirstAtVent_ClimaAutCtrldFlowReqForRowFirstAtVentLe_write) -> ClimaAutCtrldFlowReqForRowFirstAtVent_ClimaAutCtrldFlowReqForRowFirstAtVentLe/1
  ClimaMgrForVentAndFlrDistbn_Runnable_sys/17(ClimaAutCtrldFlowReqForRowFirstAtVent_ClimaAutCtrldFlowReqForRowFirstAtVentLeMid_write) -> ClimaAutCtrldFlowReqForRowFirstAtVent_ClimaAutCtrldFlowReqForRowFirstAtVentLeMid/1
  ClimaMgrForVentAndFlrDistbn_Runnable_sys/18(ClimaAutCtrldFlowReqForRowFirstAtVent_ClimaAutCtrldFlowReqForRowFirstAtVentLeSide_write) -> ClimaAutCtrldFlowReqForRowFirstAtVent_ClimaAutCtrldFlowReqForRowFirstAtVentLeSide/1
  ClimaMgrForVentAndFlrDistbn_Runnable_sys/19(ClimaAutCtrldFlowReqForRowFirstAtVent_ClimaAutCtrldFlowReqForRowFirstAtVentRi_write) -> ClimaAutCtrldFlowReqForRowFirstAtVent_ClimaAutCtrldFlowReqForRowFirstAtVentRi/1
  ClimaMgrForVentAndFlrDistbn_Runnable_sys/20(ClimaAutCtrldFlowReqForRowFirstAtVent_ClimaAutCtrldFlowReqForRowFirstAtVentRiMid_write) -> ClimaAutCtrldFlowReqForRowFirstAtVent_ClimaAutCtrldFlowReqForRowFirstAtVentRiMid/1
  ClimaMgrForVentAndFlrDistbn_Runnable_sys/21(ClimaAutCtrldFlowReqForRowFirstAtVent_ClimaAutCtrldFlowReqForRowFirstAtVentRiSide_write) -> ClimaAutCtrldFlowReqForRowFirstAtVent_ClimaAutCtrldFlowReqForRowFirstAtVentRiSide/1
  ClimaMgrForVentAndFlrDistbn_Runnable_sys/22(ClimaAutCtrldFlowReqForRowSecAtFlr_ClimaAutCtrldFlowReqForRowSecAtFlr_write) -> ClimaAutCtrldFlowReqForRowSecAtFlr_ClimaAutCtrldFlowReqForRowSecAtFlr/1
  ClimaMgrForVentAndFlrDistbn_Runnable_sys/23(ClimaAutCtrldFlowReqForRowSecAtFlr_ClimaAutCtrldFlowReqForRowSecAtFlrLe_write) -> ClimaAutCtrldFlowReqForRowSecAtFlr_ClimaAutCtrldFlowReqForRowSecAtFlrLe/1
  ClimaMgrForVentAndFlrDistbn_Runnable_sys/24(ClimaAutCtrldFlowReqForRowSecAtFlr_ClimaAutCtrldFlowReqForRowSecAtFlrRi_write) -> ClimaAutCtrldFlowReqForRowSecAtFlr_ClimaAutCtrldFlowReqForRowSecAtFlrRi/1
  ClimaMgrForVentAndFlrDistbn_Runnable_sys/25(ClimaAutCtrldFlowReqForRowSecAtVent_ClimaAutCtrldFlowReqForRowSecAtVent_write) -> ClimaAutCtrldFlowReqForRowSecAtVent_ClimaAutCtrldFlowReqForRowSecAtVent/1
  ClimaMgrForVentAndFlrDistbn_Runnable_sys/26(ClimaAutCtrldFlowReqForRowSecAtVent_ClimaAutCtrldFlowReqForRowSecAtVentLe_write) -> ClimaAutCtrldFlowReqForRowSecAtVent_ClimaAutCtrldFlowReqForRowSecAtVentLe/1
  ClimaMgrForVentAndFlrDistbn_Runnable_sys/27(ClimaAutCtrldFlowReqForRowSecAtVent_ClimaAutCtrldFlowReqForRowSecAtVentRi_write) -> ClimaAutCtrldFlowReqForRowSecAtVent_ClimaAutCtrldFlowReqForRowSecAtVentRi/1
  ClimaMgrForVentAndFlrDistbn_Runnable_sys/28(ClimaAutCtrldFlowReqForRowSecAtVentNoLim_ClimaAutCtrldFlowReqForRowSecAtVentNoLim_write) -> ClimaAutCtrldFlowReqForRowSecAtVentNoLim_ClimaAutCtrldFlowReqForRowSecAtVentNoLim/1
  ClimaMgrForVentAndFlrDistbn_Runnable_sys/29(HvacFlrLeakReqForRowFirst_HvacFlrLeakReqForRowFirst_write) -> HvacFlrLeakReqForRowFirst_HvacFlrLeakReqForRowFirst/1
  ClimaMgrForVentAndFlrDistbn_Runnable_sys/30(HvacFlrLeakReqForRowFirstLe_HvacFlrLeakReqForRowFirstLe_write) -> HvacFlrLeakReqForRowFirstLe_HvacFlrLeakReqForRowFirstLe/1
  ClimaMgrForVentAndFlrDistbn_Runnable_sys/31(HvacFlrLeakReqForRowFirstPredForStaby_HvacFlrLeakReqForRowFirstPredForStaby_write) -> HvacFlrLeakReqForRowFirstPredForStaby_HvacFlrLeakReqForRowFirstPredForStaby/1
  ClimaMgrForVentAndFlrDistbn_Runnable_sys/32(HvacFlrLeakReqForRowFirstRi_HvacFlrLeakReqForRowFirstRi_write) -> HvacFlrLeakReqForRowFirstRi_HvacFlrLeakReqForRowFirstRi/1
  ClimaMgrForVentAndFlrDistbn_Runnable_sys/33(HvacFlrLeakReqForRowSec_HvacFlrLeakReqForRowSec_write) -> HvacFlrLeakReqForRowSec_HvacFlrLeakReqForRowSec/1
  ClimaMgrForVentAndFlrDistbn_Runnable_sys/34(HvacFlrLeakReqForRowSecLe_HvacFlrLeakReqForRowSecLe_write) -> HvacFlrLeakReqForRowSecLe_HvacFlrLeakReqForRowSecLe/1
  ClimaMgrForVentAndFlrDistbn_Runnable_sys/35(HvacFlrLeakReqForRowSecPredForStaby_HvacFlrLeakReqForRowSecPredForStaby_write) -> HvacFlrLeakReqForRowSecPredForStaby_HvacFlrLeakReqForRowSecPredForStaby/1
  ClimaMgrForVentAndFlrDistbn_Runnable_sys/36(HvacFlrLeakReqForRowSecRi_HvacFlrLeakReqForRowSecRi_write) -> HvacFlrLeakReqForRowSecRi_HvacFlrLeakReqForRowSecRi/1
  ClimaMgrForVentAndFlrDistbn_Runnable_sys/37(NotAllwdForMaxReqReqdForRowSecAtVent_NotAllwdForMaxReqReqdForRowSecAtVent_write) -> NotAllwdForMaxReqReqdForRowSecAtVent_NotAllwdForMaxReqReqdForRowSecAtVent/1
  ClimaMgrForVentAndFlrDistbn_Runnable_sys/38(TModCtrlAtRowFirst_TModCtrlAtRowFirst_write) -> TModCtrlAtRowFirst_TModCtrlAtRowFirst/1
  ClimaMgrForVentAndFlrDistbn_Runnable_sys/39(TModCtrlAtRowFirst_TModCtrlAtRowFirstLe_write) -> TModCtrlAtRowFirst_TModCtrlAtRowFirstLe/1
  ClimaMgrForVentAndFlrDistbn_Runnable_sys/40(TModCtrlAtRowFirst_TModCtrlAtRowFirstRi_write) -> TModCtrlAtRowFirst_TModCtrlAtRowFirstRi/1
  ClimaMgrForVentAndFlrDistbn_Runnable_sys/41(TModCtrlAtRowSec_TModCtrlAtRowSec_write) -> TModCtrlAtRowSec_TModCtrlAtRowSec/1
  ClimaMgrForVentAndFlrDistbn_Runnable_sys/42(TModCtrlAtRowSec_TModCtrlAtRowSecLe_write) -> TModCtrlAtRowSec_TModCtrlAtRowSecLe/1
  ClimaMgrForVentAndFlrDistbn_Runnable_sys/43(TModCtrlAtRowSec_TModCtrlAtRowSecRi_write) -> TModCtrlAtRowSec_TModCtrlAtRowSecRi/1
  ClimaMgrForVentAndFlrDistbn_Runnable_sys/44(VentAndFlrModelForRowFirstLeForModelDial_VentAndFlrModelForRowFirstLeForModelDial_write) -> VentAndFlrModelForRowFirstLeForModelDial_VentAndFlrModelForRowFirstLeForModelDial/1
  ClimaMgrForVentAndFlrDistbn_Runnable_sys/45(VentAndFlrModelForRowFirstRiForModelDial_VentAndFlrModelForRowFirstRiForModelDial_write) -> VentAndFlrModelForRowFirstRiForModelDial_VentAndFlrModelForRowFirstRiForModelDial/1
  ClimaMgrForVentAndFlrDistbn_Runnable_sys/46(VentAndFlrModelForRowSecLeForModelDial_VentAndFlrModelForRowSecLeForModelDial_write) -> VentAndFlrModelForRowSecLeForModelDial_VentAndFlrModelForRowSecLeForModelDial/1
  ClimaMgrForVentAndFlrDistbn_Runnable_sys/47(VentAndFlrModelForRowSecRiForModelDial_VentAndFlrModelForRowSecRiForModelDial_write) -> VentAndFlrModelForRowSecRiForModelDial_VentAndFlrModelForRowSecRiForModelDial/1
  ClimaMgrForVentAndFlrDistbn_Runnable/1 -> ClimaMgrForVentAndFlrDistbn_Runnable_sys/Trigger

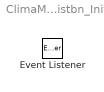
== system ClimaMgrForVentAndFlrDistbn/ClimaMgrForVentAndFlrDistbn_Init ==
  Event Listener : EventListener  EventName=reset
